simulink model "sma_corr"  (Simulink 8.0)
settings: Solver=VariableStepDiscrete  SolverMode=SingleTasking  StartTime=0.0  StopTime=1000  RelTol=1e-3
PreLoadFcn:
  | % Set this to 1 if simulating
  | simulating = 0;
  | 
  | % Overall parameters
  | n_comp = 128;
  | 
  | % Simulation
  | if (simulating)
  |     n_tvg_frames = 4;
  |     frames_per_vector = 4*8;
  |     vectors_per_wstep = 2;
  |     total_wsteps = 1; % 2^n
  |     % Laura's params
  |     x_ctr = 12;
  |     x_data_ctr = x_ctr-5;
  |     rx_addr_ctr = x_data_ctr+2;
  |     tx_addr_ctr = x_data_ctr+2;
  | else
  |     n_tvg_frames = 256;
  |     frames_per_vector = 256*8;
  |     vectors_per_wstep = 11;
  |     total_wsteps = 6; % 2^n
  |     % Laura's params
  |     x_ctr = 32;
  |     x_data_ctr = 18;
  |     rx_addr_ctr = x_data_ctr+2;
  |     tx_addr_ctr = x_data_ctr+2;
  | end
  | 
  | sb_states = repmat([ones(1, 256) zeros(1, 256)], 1, 2^(total_wsteps-1));
InitFcn:
  | % Get the XSG object to find the clock source
  | xps_xsg_blks = find_system(gcs,'FollowLinks','on','LookUnderMasks','all', 'SearchDepth',1, 'Tag','xps:xsg');
  | xsg_obj = xps_block(xps_xsg_blks{1}, {});
  | xsg_obj = xps_xsg(xsg_obj);
  | clk_src = get(xsg_obj, 'clk_src');
  | if strcmp(clk_src, 'adc0_clk')
  |     adc_brd = 'ZDOK 0';
  | elseif strcmp(clk_src, 'adc1_clk')
  |     adc_brd = 'ZDOK 1';
  | else
  |     adc_brd = '';
  | end
  | 
  | adc_clk_rate = 0;
  | % Get the appropriate ADC5g object
  | xps_adc5g_blks = find_system(gcs, 'FollowLinks', 'on', 'LookUnderMasks','all', 'SearchDepth',2, 'Tag','xps:adc5g');
  | for n = 1:length(xps_adc5g_blks)
  |     adc5g_blk = xps_adc5g_blks{n};
  |     if strcmp(get_param(adc5g_blk, 'adc_brd'), adc_brd)
  |         adc_clk_rate = 2*str2num(get_param(adc5g_blk, 'adc_clk_rate'));
  |     end
  | end
undefined symbols (not defined in the file or in its folder): Down, sfp, Up, X, xlipgetpartsetting, Z
libraries: xbsIndex_r4 x9231, casper_library_misc x178, dspsigops x32, casper_library_delays x26, xps_library x21
(part 8 of 92)

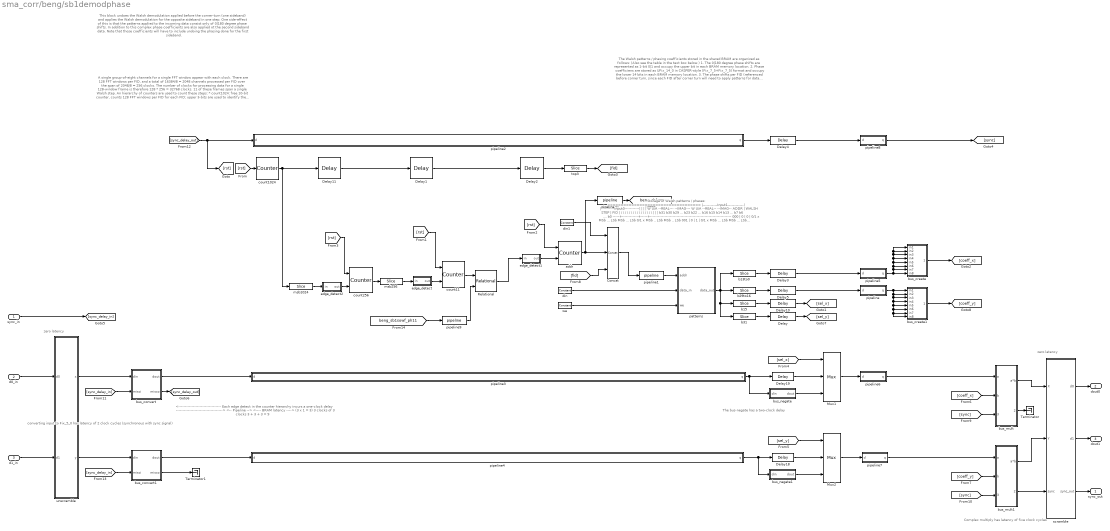
== system sma_corr/beng/sb1demodphase ==
  note: Storage of Walsh patterns / phases:
      | ===================================
      |
      |                           |-----------Input1------------| |----------Input0-----------|
      |      |            |     |  W  U/A  --REAL---   --IMAG---   W  U/A  --REAL--   --IMAG--
      | ADDR | WALSH STEP | FID | / \ / \ /         \ /         \ / \ / \ /        \ /        \
      |      |            |     | b31 b30 b29 ... b23 b22 ... b16 b15 b14 b13 ... b7 b6 .... b0
      | -----+------------+-----+-------------------------------------------------------------
      |  000 |          0 |   0 | 0/1   x MSb ... LSb MSb ... LSb 0/1   x MSb .. LSb MSb .. LSb
      |  001 |          0 |   1 | 0/1   x MSb ... LSb MSb ... LSb 0/1   x MSb .. LSb MSb .. LSb
      |    : |          : |   : |   :   :      :           :       :    :      :          :
      |  007 |          0 |   7 | 0/1   x MSb ... LSb MSb ... LSb 0/1   x MSb .. LSb MSb .. LSb
      |  008 |          1 |   0 | 0/1   x MSb ... LSb MSb ... LSb 0/1   x MSb .. LSb MSb .. LSb
      |  009 |          1 |   2 | 0/1   x MSb ... LSb MSb ... LSb 0/1   x MSb .. LSb MSb .. LSb
      |    : |          : |   : |   :   :      :           :       :    :      :          :
      |  1FF |         63 |   7 | 0/1   x MSb ... LSb MSb ... LSb 0/1   x MSb .. LSb MSb .. LSb
      |  200 |        N/A | N/A |   x   x      x           x       x    x      x          x
      |  201 |        N/A | N/A |   x   x      x           x       x    x      x          x
      |    : |          : |   : |   :   :      :           :       :    :      :          :
      |  3FF |        N/A | N/A |   x   x      x           x       x    x      x          x
  note: A single group-of-eight channels for a single FFT window appear with each clock. There are 128 FFT windows per FID,
      | and a total of 16384/8 = 2048 channels processed per FID over the span of 2048/8 = 256 clocks. The number of
      | clocks for processing data for a single 128-window frame is therefore 128 * 256 = 32768 clocks. 11 of these frames
      | span a single Walsh step. An hierarchy of counters are used to count these steps:
      |
      | 	* count1024: free 10-bit counter, counts 128 FFT windows per FID for each FID; upper 3-bits are used to identify
      | 	the current FID to select the correct bit from the loaded Walsh pattern
      |
      | 	* count256: free 8-bit counter, increments for each wrap of count1024; counts 256 group-of-eights, so when this
      | 	counter wraps, a complete 128-window frame has been processed
      |
      | 	* count11: count-limited 4-bit counter, increments for each wrap of count256; counts the 11 frames that span a
      | 	single Walsh step
      |
      | 	* addr: free 6-bit counter, increments for each wrap of count11; counts the Walsh steps in a Walsh frame; the
      | 	output is used to address the current Walsh phase that need to be applied to all FIDs.
  note: The Walsh patterns / phasing coefficients stored in the shared BRAM are organized as follows:
      | (Also see the table in the text box below.)
      |
      | 	1. The 0|180 degree phase shifts are represented as 1-bit 0|1 and occupy the upper bit in
      | 	each BRAM memory location.
      |
      | 	2. Phase coefficiens are stored as UFix_14_0 in CASPER-style (Fix_7_5+Fix_7_5) format and
      | 	occupy the lower 14 bits in each BRAM memory location.
      |
      | 	3. The phase shifts per FID (referenced before corner turn, since each FID after corner turn
      | 	will need to apply patterns for data digitized and demodulated in ALL FIDs before corner
      | 	turn) for a single Walsh step is stored in 8 consecutive shared BRAM entries along with the
      | 	phasing coefficients for each FID.
      |
      | 	4. The patterns for 64 Walsh steps are stored in sequentially blocks of eight addresses in
      | 	BRAM, so that the patterns occupy the upper bits in 64x8 = 512 (0x000-0x1FF) memory
      | 	locations. The phasing coefficients stored in memory should repeat with every Walsh step
      | 	so constant phasing is applied over the length of a complete Walsh frame.
      |
      | 	5. Due to toolflow constraints a	shared BRAM with 16-bit data has to have an address width
      | 	of at least 11 bits so the upper three quarters of memoryspace is unused.
  note: This block undoes the Walsh demodulation applied before the corner-turn (one sideband) and
      | applies the Walsh demodulation for the opposite sideband in one step. One side-effect of this
      | is that the patterns applied to the incoming data consist only of 0|180 degree phase shifts.
      |
      | In addition to this complex phase coefficients are also applied ot the second sideband data.
      | Note that those coefficients will have to include undoing the phasing done for the first
      | sideband.
  note: <-------------------------------- Each edge detect in the counter hierarchy incurs a one-clock delay -----------------------------------> <-- Pipeline --> <---- BRAM latency ---->
      |                                                     (3 x 1 = 3)                                                                               3 clocks           of 3 clocks
      |                                                            3                                                                             +        3       +           3
      |                                                          = 9
  note: The bus-negate has a
      | two-clock delay
  note: Complex multiply has latency
      | of five clock  cycles
  note: converting input to Fix_5_0 has latency
      | of 2 clock cycles (synchronous with sync
      | signal)
  note: zero latency
  note: zero latency
  sync_in : Inport
  d0_in : Inport  Port=2
  d1_in : Inport  Port=3
  Concat : xbsIndex_r4/Concat  num_inputs=3  dbl_ovrd=off  block_type=concat
  Delay{,3..5,10,18..19} (7 alike) : xbsIndex_r4/Delay  rst=off  infoeditControl=Selection of Reset will increase slice count due to use of real FFs and instead of SRLs  en=off  latency=2  dbl_ovrd=off  reg_retiming=off  block_type=delay
  Delay1 : xbsIndex_r4/Delay  rst=off  infoeditControl=Selection of Reset will increase slice count due to use of real FFs and instead of SRLs  en=off  latency=1  dbl_ovrd=off  reg_retiming=off  block_type=delay
  Delay11 : xbsIndex_r4/Delay  rst=off  infoeditControl=Selection of Reset will increase slice count due to use of real FFs and instead of SRLs  en=off  latency=1  dbl_ovrd=off  reg_retiming=off  block_type=delay
  Delay2 : xbsIndex_r4/Delay  rst=off  infoeditControl=Selection of Reset will increase slice count due to use of real FFs and instead of SRLs  en=off  latency=1  dbl_ovrd=off  reg_retiming=off  block_type=delay
  Mux1 : xbsIndex_r4/Mux  inputs=2  en=off  latency=0  precision=Full  arith_type=Unsigned  n_bits=16  bin_pt=14  quantization=Truncate  overflow=Wrap  dbl_ovrd=off  block_type=mux
  Mux2 : xbsIndex_r4/Mux  inputs=2  en=off  latency=0  precision=Full  arith_type=Unsigned  n_bits=16  bin_pt=14  quantization=Truncate  overflow=Wrap  dbl_ovrd=off  block_type=mux
  Relational : xbsIndex_r4/Relational  mode=a=b  en=off  latency=0  dbl_ovrd=off  block_type=relational
  Terminator : Terminator
  Terminator1 : Terminator
  addr : xbsIndex_r4/Counter  cnt_type=Free Running  cnt_to=Inf  operation=Up  start_count=0  cnt_by_val=1  arith_type=Unsigned  n_bits=6  bin_pt=0  load_pin=off  rst=on  en=on  explicit_period=on  period=1  dbl_ovrd=off  use_behavioral_HDL=off  implementation=Fabric  block_type=counter
  b13to0 : xbsIndex_r4/Slice  nbits=14  boolean_output=off  mode=Lower Bit Location + Width  bit1=16  base1=LSB of Input  bit0=0  base0=LSB of Input  dbl_ovrd=off  block_type=slice
  b15 : xbsIndex_r4/Slice  nbits=1  boolean_output=off  mode=Lower Bit Location + Width  bit1=0  base1=MSB of Input  bit0=15  base0=LSB of Input  dbl_ovrd=off  block_type=slice
  b29to16 : xbsIndex_r4/Slice  nbits=14  boolean_output=off  mode=Lower Bit Location + Width  bit1=0  base1=MSB of Input  bit0=16  base0=LSB of Input  dbl_ovrd=off  block_type=slice
  b31 : xbsIndex_r4/Slice  nbits=1  boolean_output=off  mode=Lower Bit Location + Width  bit1=0  base1=MSB of Input  bit0=31  base0=LSB of Input  dbl_ovrd=off  block_type=slice
  bus_convert : Subsystem  AncestorBlock=casper_library_bus/bus_convert  mask(bus_convert){n_bits_in=[4 4 4 4 4 4 4 4], bin_pt_in=[0 0 0 0 0 0 0 0], cmplx=on, n_bits_out=[5 5 5 5 5 5 5 5], bin_pt_out=[0 0 0 0 0 0 0 0], quantization=1, overflow=1, latency=2, of=off, misc=on}
      mask prompts: n_bits_in "input bit widths"; bin_pt_in "input binary points"; cmplx "input complex"; n_bits_out "output bit widths"; bin_pt_out "output binary points"; quantization "quantization strategy (Truncate=0, Round  (unbiased: +/- Inf)=1, Round (unbiased: Even Values)=2)"; overflow "overflow strategy (Wrap=0, Saturate=1, Flag as error=2)"; of "overflow indication"; misc "misc support"
      mask help: Convert components of busses
      mask init: same as sma_corr/fengine_0/cnv
  bus_convert1 : Subsystem  AncestorBlock=casper_library_bus/bus_convert  mask(bus_convert){n_bits_in=[4 4 4 4 4 4 4 4], bin_pt_in=[0 0 0 0 0 0 0 0], cmplx=on, n_bits_out=[5 5 5 5 5 5 5 5], bin_pt_out=[0 0 0 0 0 0 0 0], quantization=1, overflow=1, latency=2, of=off, misc=on}
      mask prompts: n_bits_in "input bit widths"; bin_pt_in "input binary points"; cmplx "input complex"; n_bits_out "output bit widths"; bin_pt_out "output binary points"; quantization "quantization strategy (Truncate=0, Round  (unbiased: +/- Inf)=1, Round (unbiased: Even Values)=2)"; overflow "overflow strategy (Wrap=0, Saturate=1, Flag as error=2)"; of "overflow indication"; misc "misc support"
      mask help: Convert components of busses
      mask init: same as sma_corr/fengine_0/cnv
  bus_create : Subsystem  AncestorBlock=casper_library_flow_control/bus_create  mask(bus_create){inputNum=8}
      mask prompts: inputNum "Number of inputs:"
      mask init:
      | bus_create_init(gcb,...
      |     'inputNum', inputNum);
  bus_create1 : Subsystem  AncestorBlock=casper_library_flow_control/bus_create  mask(bus_create){inputNum=8}
      mask prompts: inputNum "Number of inputs:"
      mask init:
      | bus_create_init(gcb,...
      |     'inputNum', inputNum);
  bus_mult : Subsystem  AncestorBlock=casper_library_bus/bus_mult  mask(bus_mult){n_bits_a=[5 5 5 5 5 5 5 5], bin_pt_a=[0 0 0 0 0 0 0 0], type_a=[1 1 1 1 1 1 1 1], cmplx_a=on, n_bits_b=[7 7 7 7 7 7 7 7], bin_pt_b=[5 5 5 5 5 5 5 5], type_b=[1 1 1 1 1 1 1 1], cmplx_b=on, n_bits_out=[1 1 1 1 1 1 1 1]*8, bin_pt_out=[1 1 1 1 1 1 1 1]*4, type_out=[1 1 1 1 1 1 1 1], quantization=0, overflow=1, mult_latency=3, add_latency=1, conv_latency=1, max_fanout=2, fan_latency=0, multiplier_implementation=standard core, misc=on}
      mask prompts: n_bits_a "a input bit widths"; bin_pt_a "a input binary points"; type_a "a input type (Unsigned=0, Signed=1)"; cmplx_a "a input complex"; n_bits_b "b input bit widths"; bin_pt_b "b input binary points"; type_b "b input type (Unsigned=0, Signed=1)"; cmplx_b "b input complex"; n_bits_out "output bit widths"; bin_pt_out "output binary points"; type_out "output type (Unsigned=0, Signed=1)"; quantization "quantization strategy (Truncate=0, Round  (unbiased: +/- Inf)=1)"; overflow "overflow strategy (Wrap=0, Saturate=1, Flag as error=2)"; mult_latency "multiplier latency"; add_latency "adder latency"; conv_latency "convert latency"; max_fanout "limit fanout to ?"; fan_latency "fanout register latency"; multiplier_implementation "multiplier implementation"; misc "misc support"
      mask help: Multiply components of two busses
      mask init:
      | bus_mult_init(gcb, ...
      |     'n_bits_a', n_bits_a, ...
      |     'bin_pt_a', bin_pt_a, ...
      |     'type_a', type_a, ...
      |     'cmplx_a', cmplx_a, ...
      |     'n_bits_b', n_bits_b, ...
      |     'bin_pt_b', bin_pt_b, ...
      |     'type_b', type_b, ...
      |     'cmplx_b', cmplx_b, ...
      |     'n_bits_out', n_bits_out, ...
      |     'bin_pt_out', bin_pt_out, ...
      |     'type_out', type_out, ...
      |     'quantization', quantization, ...
      |     'overflow', overflow, ...
      |     'mult_latency', mult_latency, ...
      |     'add_latency', add_latency, ...
      |     'conv_latency', conv_latency, ...
      |     'max_fanout', max_fanout, ...
      |     'fan_latency', fan_latency, ...
      |     'multiplier_implementation', multiplier_implementation, ...
      |     'misc', misc);
      mask TypeOptions multiplier_implementation: behavioral HDL | standard core | embedded multiplier core
  bus_mult1 : Subsystem  AncestorBlock=casper_library_bus/bus_mult  mask(bus_mult){n_bits_a=[5 5 5 5 5 5 5 5], bin_pt_a=[0 0 0 0 0 0 0 0], type_a=[1 1 1 1 1 1 1 1], cmplx_a=on, n_bits_b=[7 7 7 7 7 7 7 7], bin_pt_b=[5 5 5 5 5 5 5 5], type_b=[1 1 1 1 1 1 1 1], cmplx_b=on, n_bits_out=[1 1 1 1 1 1 1 1]*8, bin_pt_out=[1 1 1 1 1 1 1 1]*4, type_out=[1 1 1 1 1 1 1 1], quantization=0, overflow=1, mult_latency=3, add_latency=1, conv_latency=1, max_fanout=2, fan_latency=0, multiplier_implementation=standard core, misc=on}
      mask prompts: n_bits_a "a input bit widths"; bin_pt_a "a input binary points"; type_a "a input type (Unsigned=0, Signed=1)"; cmplx_a "a input complex"; n_bits_b "b input bit widths"; bin_pt_b "b input binary points"; type_b "b input type (Unsigned=0, Signed=1)"; cmplx_b "b input complex"; n_bits_out "output bit widths"; bin_pt_out "output binary points"; type_out "output type (Unsigned=0, Signed=1)"; quantization "quantization strategy (Truncate=0, Round  (unbiased: +/- Inf)=1)"; overflow "overflow strategy (Wrap=0, Saturate=1, Flag as error=2)"; mult_latency "multiplier latency"; add_latency "adder latency"; conv_latency "convert latency"; max_fanout "limit fanout to ?"; fan_latency "fanout register latency"; multiplier_implementation "multiplier implementation"; misc "misc support"
      mask help: Multiply components of two busses
      mask init: same as sma_corr/beng/sb1demodphase/bus_mult
      mask TypeOptions multiplier_implementation: behavioral HDL | standard core | embedded multiplier core
  bus_negate : Subsystem  AncestorBlock=casper_library_bus/bus_negate  mask(bus_negate){n_bits_in=[5 5 5 5 5 5 5 5], bin_pt_in=0, cmplx=on, overflow=1, latency=2, misc=off}
      mask prompts: n_bits_in "input bit widths"; bin_pt_in "input binary points"; cmplx "input complex"; overflow "overflow strategy (Wrap=0, Saturate=1, Flag as error=2)"; misc "misc support"
      mask help: Negate components of busses
      mask init:
      | bus_negate_init(gcb, ...
      |     'n_bits_in', n_bits_in, ...
      |     'bin_pt_in', bin_pt_in, ...
      |     'cmplx', cmplx, ...
      |     'overflow', overflow, ...
      |     'latency', latency, ...
      |     'misc', misc);
  bus_negate1 : Subsystem  AncestorBlock=casper_library_bus/bus_negate  mask(bus_negate){n_bits_in=[5 5 5 5 5 5 5 5], bin_pt_in=0, cmplx=on, overflow=1, latency=2, misc=off}
      mask prompts: n_bits_in "input bit widths"; bin_pt_in "input binary points"; cmplx "input complex"; overflow "overflow strategy (Wrap=0, Saturate=1, Flag as error=2)"; misc "misc support"
      mask help: Negate components of busses
      mask init:
      | bus_negate_init(gcb, ...
      |     'n_bits_in', n_bits_in, ...
      |     'bin_pt_in', bin_pt_in, ...
      |     'cmplx', cmplx, ...
      |     'overflow', overflow, ...
      |     'latency', latency, ...
      |     'misc', misc);
  count1024 : xbsIndex_r4/Counter  cnt_type=Free Running  cnt_to=Inf  operation=Up  start_count=0  cnt_by_val=1  arith_type=Unsigned  n_bits=10  bin_pt=0  load_pin=off  rst=on  en=off  explicit_period=on  period=1  dbl_ovrd=off  use_behavioral_HDL=off  implementation=Fabric  block_type=counter
  count11 : xbsIndex_r4/Counter  cnt_type=Count Limited  cnt_to=10  operation=Up  start_count=0  cnt_by_val=1  arith_type=Unsigned  n_bits=4  bin_pt=0  load_pin=off  rst=on  en=on  explicit_period=on  period=1  dbl_ovrd=off  use_behavioral_HDL=off  implementation=Fabric  block_type=counter
  count256 : xbsIndex_r4/Counter  cnt_type=Free Running  cnt_to=10  operation=Up  start_count=0  cnt_by_val=1  arith_type=Unsigned  n_bits=8  bin_pt=0  load_pin=off  rst=on  en=on  explicit_period=on  period=1  dbl_ovrd=off  use_behavioral_HDL=off  implementation=Fabric  block_type=counter
  din : xbsIndex_r4/Constant  const=0  gui_display_data_type=Fixed-point  arith_type=Unsigned  n_bits=8  bin_pt=0  preci_type=Single  exp_width=8  frac_width=24  explicit_period=on  period=1  equ=P=C  opselect=C  inp2=PCIN>>17  opr=+  inp1=P  carry=CIN  dbl_ovrd=off  block_type=constant
  din1 : xbsIndex_r4/Constant  const=0  gui_display_data_type=Fixed-point  arith_type=Unsigned  n_bits=1  bin_pt=0  preci_type=Single  exp_width=8  frac_width=24  explicit_period=on  period=1  equ=P=C  opselect=C  inp2=PCIN>>17  opr=+  inp1=P  carry=CIN  dbl_ovrd=off  block_type=constant
  edge_detect : Subsystem  AncestorBlock=casper_library_misc/edge_detect  mask(edge_detect){edge=Falling, polarity=Active High, x_in=[0 0.08 0.08 0.16 0.16 0.24 0.24 0.32 0.32 0.4], y_in=[-1 -1  1  1  1  1  1  1  1  1], x_out=[0.6 0.68 0.68 0.76 0.76 0.84 0.84 0.92 0.92 1], y_out=[-1 -1  1  1 -1 -1 -1 -1 -1 -1]}
      mask prompts: edge "Edge Type"; polarity "Output Polarity"; x_in "Input X Positions"; y_in "Input Y Positions"; x_out "Output X Positions"; y_out "Output Y Positions"
      mask help: Output is active for one-cycle after each edge of the specified type (i.e. rising, falling or both).
      mask init: same as sma_corr/scope/edge_detect
      settings: AttributesFormatString=|
      | falling edges
      | active high
      mask Help: Output is active for one-cycle after each edge of the specified type (i.e. rising, falling, or both).  Latency is zero.
      mask TypeOptions edge: Rising | Falling | Both
      mask TypeOptions polarity: Active High | Active Low
      mask Display:
      | plot(x_in_num,0.5+y_in_num*0.2);
      | plot(x_out_num,0.5+y_out_num*0.2);
  edge_detect1 : Subsystem  AncestorBlock=casper_library_misc/edge_detect  mask(edge_detect){edge=Falling, polarity=Active High, x_in=[0 0.08 0.08 0.16 0.16 0.24 0.24 0.32 0.32 0.4], y_in=[-1 -1  1  1  1  1  1  1  1  1], x_out=[0.6 0.68 0.68 0.76 0.76 0.84 0.84 0.92 0.92 1], y_out=[-1 -1  1  1 -1 -1 -1 -1 -1 -1]}
      mask prompts: edge "Edge Type"; polarity "Output Polarity"; x_in "Input X Positions"; y_in "Input Y Positions"; x_out "Output X Positions"; y_out "Output Y Positions"
      mask help: Output is active for one-cycle after each edge of the specified type (i.e. rising, falling or both).
      mask init: same as sma_corr/scope/edge_detect
      settings: AttributesFormatString=|
      | falling edges
      | active high
      mask Help: Output is active for one-cycle after each edge of the specified type (i.e. rising, falling, or both).  Latency is zero.
      mask TypeOptions edge: Rising | Falling | Both
      mask TypeOptions polarity: Active High | Active Low
      mask Display:
      | plot(x_in_num,0.5+y_in_num*0.2);
      | plot(x_out_num,0.5+y_out_num*0.2);
  edge_detect2 : Subsystem  AncestorBlock=casper_library_misc/edge_detect  mask(edge_detect){edge=Falling, polarity=Active High, x_in=[0 0.08 0.08 0.16 0.16 0.24 0.24 0.32 0.32 0.4], y_in=[-1 -1  1  1  1  1  1  1  1  1], x_out=[0.6 0.68 0.68 0.76 0.76 0.84 0.84 0.92 0.92 1], y_out=[-1 -1  1  1 -1 -1 -1 -1 -1 -1]}
      mask prompts: edge "Edge Type"; polarity "Output Polarity"; x_in "Input X Positions"; y_in "Input Y Positions"; x_out "Output X Positions"; y_out "Output Y Positions"
      mask help: Output is active for one-cycle after each edge of the specified type (i.e. rising, falling or both).
      mask init: same as sma_corr/scope/edge_detect
      settings: AttributesFormatString=|
      | falling edges
      | active high
      mask Help: Output is active for one-cycle after each edge of the specified type (i.e. rising, falling, or both).  Latency is zero.
      mask TypeOptions edge: Rising | Falling | Both
      mask TypeOptions polarity: Active High | Active Low
      mask Display:
      | plot(x_in_num,0.5+y_in_num*0.2);
      | plot(x_out_num,0.5+y_out_num*0.2);
  msb1024 : xbsIndex_r4/Slice  nbits=1  boolean_output=on  mode=Upper Bit Location + Width  bit1=0  base1=MSB of Input  bit0=0  base0=LSB of Input  dbl_ovrd=off  block_type=slice
  msb256 : xbsIndex_r4/Slice  nbits=1  boolean_output=on  mode=Upper Bit Location + Width  bit1=0  base1=MSB of Input  bit0=0  base0=LSB of Input  dbl_ovrd=off  block_type=slice
  patterns : Subsystem  Tag=xps:bram  AncestorBlock=xps_library/Shared BRAM  mask{arith_type=Unsigned, addr_width=10, data_width=32, reg_prim_output=on, reg_core_output=on, optimization=Minimum_Area, data_bin_pt=0, init_vals=ones(1,1024)*2101248, sample_rate=1}
      mask prompts: arith_type "Output Data Type"; addr_width "Address width"; data_width "Data Width"; reg_prim_output "Register Primitive Output"; reg_core_output "Register Core Output"; data_bin_pt "Data Binary Point"; init_vals "Initial values (simulation only)"; sample_rate "Sample rate"
      mask init:
      | shared_bram_mask;
      mask TypeOptions arith_type: Unsigned | Signed  (2's comp)
      mask TypeOptions data_width: 8 | 16 | 32 | 64 | 128
      mask TypeOptions optimization: Minimum_Area | Low_Power
  pipeline : Subsystem  AncestorBlock=casper_library_delays/pipeline  mask(pipeline){latency=5}
      mask help:
      | An explicitly laid-out delay line to avoid mapping to SRL16s
      | for use in pipelining to help achieve timing closure.
      mask init:
      | pipeline_init(gcb,'latency',latency);
      settings: AttributesFormatString=Latency = %<latency>
      mask Display:
      | port_label('input', 1, 'd');
      | port_label('output', 1, 'q');
      | disp(['Z^-', num2str(latency)]);
  pipeline1 : casper_library_delays/pipeline  latency=3
      settings: AttributesFormatString=Latency = %<latency>
  pipeline10 : casper_library_delays/pipeline  latency=3
      settings: AttributesFormatString=Latency = %<latency>
  pipeline2 : Subsystem  AncestorBlock=casper_library_delays/pipeline  mask(pipeline){latency=9}
      mask help:
      | An explicitly laid-out delay line to avoid mapping to SRL16s
      | for use in pipelining to help achieve timing closure.
      mask init:
      | pipeline_init(gcb,'latency',latency);
      settings: AttributesFormatString=Latency = %<latency>
      mask Display:
      | port_label('input', 1, 'd');
      | port_label('output', 1, 'q');
      | disp(['Z^-', num2str(latency)]);
  pipeline3 : Subsystem  AncestorBlock=casper_library_delays/pipeline  mask(pipeline){latency=9}
      mask help:
      | An explicitly laid-out delay line to avoid mapping to SRL16s
      | for use in pipelining to help achieve timing closure.
      mask init:
      | pipeline_init(gcb,'latency',latency);
      settings: AttributesFormatString=Latency = %<latency>
      mask Display:
      | port_label('input', 1, 'd');
      | port_label('output', 1, 'q');
      | disp(['Z^-', num2str(latency)]);
  pipeline4 : Subsystem  AncestorBlock=casper_library_delays/pipeline  mask(pipeline){latency=9}
      mask help:
      | An explicitly laid-out delay line to avoid mapping to SRL16s
      | for use in pipelining to help achieve timing closure.
      mask init:
      | pipeline_init(gcb,'latency',latency);
      settings: AttributesFormatString=Latency = %<latency>
      mask Display:
      | port_label('input', 1, 'd');
      | port_label('output', 1, 'q');
      | disp(['Z^-', num2str(latency)]);
  pipeline5 : Subsystem  AncestorBlock=casper_library_delays/pipeline  mask(pipeline){latency=5}
      mask help:
      | An explicitly laid-out delay line to avoid mapping to SRL16s
      | for use in pipelining to help achieve timing closure.
      mask init:
      | pipeline_init(gcb,'latency',latency);
      settings: AttributesFormatString=Latency = %<latency>
      mask Display:
      | port_label('input', 1, 'd');
      | port_label('output', 1, 'q');
      | disp(['Z^-', num2str(latency)]);
  pipeline6 : Subsystem  AncestorBlock=casper_library_delays/pipeline  mask(pipeline){latency=5}
      mask help:
      | An explicitly laid-out delay line to avoid mapping to SRL16s
      | for use in pipelining to help achieve timing closure.
      mask init:
      | pipeline_init(gcb,'latency',latency);
      settings: AttributesFormatString=Latency = %<latency>
      mask Display:
      | port_label('input', 1, 'd');
      | port_label('output', 1, 'q');
      | disp(['Z^-', num2str(latency)]);
  pipeline7 : Subsystem  AncestorBlock=casper_library_delays/pipeline  mask(pipeline){latency=5}
      mask help:
      | An explicitly laid-out delay line to avoid mapping to SRL16s
      | for use in pipelining to help achieve timing closure.
      mask init:
      | pipeline_init(gcb,'latency',latency);
      settings: AttributesFormatString=Latency = %<latency>
      mask Display:
      | port_label('input', 1, 'd');
      | port_label('output', 1, 'q');
      | disp(['Z^-', num2str(latency)]);
  pipeline8 : Subsystem  AncestorBlock=casper_library_delays/pipeline  mask(pipeline){latency=5}
      mask help:
      | An explicitly laid-out delay line to avoid mapping to SRL16s
      | for use in pipelining to help achieve timing closure.
      mask init:
      | pipeline_init(gcb,'latency',latency);
      settings: AttributesFormatString=Latency = %<latency>
      mask Display:
      | port_label('input', 1, 'd');
      | port_label('output', 1, 'q');
      | disp(['Z^-', num2str(latency)]);
  pipeline9 : casper_library_delays/pipeline  latency=3
      settings: AttributesFormatString=Latency = %<latency>
  scramble : Subsystem
  top3 : xbsIndex_r4/Slice  nbits=3  boolean_output=off  mode=Upper Bit Location + Width  bit1=0  base1=MSB of Input  bit0=0  base0=LSB of Input  dbl_ovrd=off  block_type=slice
  unscramble : Subsystem
  we : xbsIndex_r4/Constant  const=0  gui_display_data_type=Fixed-point  arith_type=Unsigned  n_bits=8  bin_pt=0  preci_type=Single  exp_width=8  frac_width=24  explicit_period=on  period=1  equ=P=C  opselect=C  inp2=PCIN>>17  opr=+  inp1=P  carry=CIN  dbl_ovrd=off  block_type=constant
  sync_out : Outport
  dout0 : Outport  Port=2
  dout1 : Outport  Port=3
  tag scope: beng_sb1ws=global
  wires:
  bus_negate/1(dout) -> Mux1/3
  Delay19/1 -> Mux1/2
  b15/1 -> Delay10/1
  patterns/1(data_out) -> b13to0/1, b29to16/1, b31/1, b15/1
  msb256/1 -> edge_detect/1(in)
  edge_detect/1(out) -> count11/2
  din/1 -> patterns/2(data_in)
  we/1 -> patterns/3(we)
  count11/1 -> Relational/1
  [rst] -> count1024/1
  [rst] -> count11/1
  [rst] -> addr/1
  Delay1/1 -> Delay2/1
  pipeline3/1(q) -> bus_negate/1(din), Delay19/1
  Relational/1 -> edge_detect1/1(in)
  edge_detect1/1(out) -> addr/2
  msb1024/1 -> edge_detect2/1(in)
  edge_detect2/1(out) -> count256/2
  [rst] -> count256/1
  count256/1 -> msb256/1
  Delay11/1 -> Delay1/1
  count1024/1 -> Delay11/1, msb1024/1
  bus_negate1/1(dout) -> Mux2/3
  Delay18/1 -> Mux2/2
  pipeline4/1(q) -> Delay18/1, bus_negate1/1(din)
  Delay10/1 -> [sel_x]
  [sel_x] -> Mux1/1
  [sel_y] -> Mux2/1
  pipeline6/1(q) -> bus_mult/1(a)
  Mux1/1 -> pipeline6/1(d)
  pipeline7/1(q) -> bus_mult1/1(a)
  Mux2/1 -> pipeline7/1(d)
  bus_mult1/2(misco) -> scramble/3(sync)
  bus_create/1(bus_out) -> [coeff_x]
  [coeff_x] -> bus_mult/2(b)
  [coeff_y] -> bus_mult1/2(b)
  Delay2/1 -> top3/1
  top3/1 -> [fid]
  [fid] -> Concat/3
  addr/1 -> pipeline10/1, Concat/2
  din1/1 -> Concat/1
  b13to0/1 -> Delay3/1
  [sync] -> bus_mult/3(misci)
  [sync] -> bus_mult1/3(misci)
  Delay3/1 -> pipeline5/1(d)
  bus_convert/1(dout) -> pipeline3/1(d)
  sync_in/1 -> [sync_delay_in]
  [sync_delay_in] -> bus_convert/2(misci)
  bus_convert/2(misco) -> [sync_delay_out]
  [sync_delay_out] -> pipeline2/1(d), [rst]
  [sync_delay_in] -> bus_convert1/2(misci)
  bus_convert1/2(misco) -> Terminator1/1
  bus_convert1/1(dout) -> pipeline4/1(d)
  pipeline1/1 -> patterns/1(addr)
  Concat/1 -> pipeline1/1
  pipeline8/1(q) -> [sync]
  pipeline2/1(q) -> Delay4/1
  Delay4/1 -> pipeline8/1(d)
  d0_in/1 -> unscramble/1(d0)
  d1_in/1 -> unscramble/2(d1)
  unscramble/2(y) -> bus_convert1/1(din)
  unscramble/1(x) -> bus_convert/1(din)
  pipeline5/1(q) -> bus_create/1(in1), bus_create/2(in2), bus_create/3(in3), bus_create/4(in4), bus_create/5(in5), bus_create/6(in6), bus_create/7(in7), bus_create/8(in8)
  Delay/1 -> [sel_y]
  b31/1 -> Delay/1
  b29to16/1 -> Delay5/1
  Delay5/1 -> pipeline/1(d)
  pipeline/1(q) -> bus_create1/2(in2), bus_create1/3(in3), bus_create1/4(in4), bus_create1/5(in5), bus_create1/6(in6), bus_create1/7(in7), bus_create1/8(in8), bus_create1/1(in1)
  bus_create1/1(bus_out) -> [coeff_y]
  bus_mult/2(misco) -> Terminator/1
  bus_mult/1(a*b) -> scramble/1(X)
  bus_mult1/1(a*b) -> scramble/2(Y)
  scramble/1(d0) -> dout0/1
  scramble/2(d1) -> dout1/1
  scramble/3(sync_out) -> sync_out/1
  [beng_sb1sowf_ph11] -> pipeline9/1
  pipeline9/1 -> Relational/2
  pipeline10/1 -> [beng_sb1ws]

== system sma_corr/beng/sum0 ==
  note: din is 64 bits wide.
      | This is eight 4+4-bit samples wide.
      | More specifically, it is 4 dual-pol
      | 4+4 bit samples:
      |
      | ||CaX|CaY||CbX|CbY||CcX|CcY||CdX|CdY||
      |
      | The four summers operate in SIMD mode
      | on four 4-bit slices of data.  We want
      | to enable/disable the two polarizations
      | indpendently so each summer must process
      | data from a single polarization only.
      |
      | The input BitBasher block slices the
      | data appropriately and the output
      | BitBasher block reassembles the data
      | back to the original layout (though
      | each sample is now wider after summing).
  gain : Inport
  sync_in : Inport  Port=2
  din : Inport  Port=3
  disable : Inport  Port=4
  BitBasher : xbsIndex_r4/BitBasher  bitexpr=|  display_expr=off  sr_1=1  arith_type1=Unsigned  bin_pt1=0  sr_2=2  arith_type2=Unsigned  bin_pt2=0  sr_3=3  arith_type3=Unsigned  bin_pt3=0  sr_4=4  arith_type4=Unsigned  bin_pt4=0  dbl_ovrd=off  block_type=bitbasher
      | <!DOCTYPE HTML PUBLIC "-//W3C//DTD HTML 4.0//EN" "http://www.w3.org/TR/REC-html40/strict.dtd">
      | <html><head><meta name="qrichtext" content="1" /><style type="text/css">
      | p, li { white-space: pre-wrap; }
      | </style></head><body style=" font-family:'Sans'; font-size:10pt; font-weight:400; font-style:normal;">
      | <p style=" margin-top:0px; margin-bottom:0px; margin-left:0px; margin-right:0px; -qt-block-indent:0; text-indent:0px;"><span style=" font-family:'MS Shell Dlg'; font-size:8pt;">Xab={i[63:56], i[47:40]}<br />Xcd={i[31:24], i[15:8]}<br />Yab={i[55:48], i[39:32]}<br />Ycd={i[23:16], i[7:0]}<br /></span></p></body></html>
  BitBasher1 : xbsIndex_r4/BitBasher  bitexpr=|  display_expr=off  sr_1=1  arith_type1=Unsigned  bin_pt1=4  sr_2=2  arith_type2=Unsigned  bin_pt2=4  sr_3=3  arith_type3=Unsigned  bin_pt3=0  sr_4=4  arith_type4=Unsigned  bin_pt4=0  dbl_ovrd=off  block_type=bitbasher
      | <!DOCTYPE HTML PUBLIC "-//W3C//DTD HTML 4.0//EN" "http://www.w3.org/TR/REC-html40/strict.dtd">
      | <html><head><meta name="qrichtext" content="1" /><style type="text/css">
      | p, li { white-space: pre-wrap; }
      | </style></head><body style=" font-family:'Sans'; font-size:10pt; font-weight:400; font-style:normal;">
      | <p style=" margin-top:12px; margin-bottom:12px; margin-left:0px; margin-right:0px; -qt-block-indent:0; text-indent:0px;"><span style=" font-family:'MS Shell Dlg'; font-size:8pt;">gx={gyx[7:0]}<br />gy={gyx[15:8]}</span></p></body></html>
  BitBasher2 : xbsIndex_r4/BitBasher  bitexpr=|  display_expr=off  sr_1=1  arith_type1=Unsigned  bin_pt1=0  sr_2=2  arith_type2=Unsigned  bin_pt2=0  sr_3=3  arith_type3=Unsigned  bin_pt3=0  sr_4=4  arith_type4=Unsigned  bin_pt4=0  dbl_ovrd=off  block_type=bitbasher
      | <!DOCTYPE HTML PUBLIC "-//W3C//DTD HTML 4.0//EN" "http://www.w3.org/TR/REC-html40/strict.dtd">
      | <html><head><meta name="qrichtext" content="1" /><style type="text/css">
      | p, li { white-space: pre-wrap; }
      | </style></head><body style=" font-family:'Sans'; font-size:10pt; font-weight:400; font-style:normal;">
      | <p style=" margin-top:0px; margin-bottom:0px; margin-left:0px; margin-right:0px; -qt-block-indent:0; text-indent:0px;"><span style=" font-family:'MS Shell Dlg'; font-size:8pt;">o={Xab[15:8], Yab[15:8], Xab[7:0], Yab[7:0], Xcd[15:8], Ycd[15:8], Xcd[7:0], Ycd[7:0]}</span></p></body></html>
  BitBasher3 : xbsIndex_r4/BitBasher  bitexpr=|  display_expr=off  sr_1=1  arith_type1=Unsigned  bin_pt1=0  sr_2=2  arith_type2=Unsigned  bin_pt2=0  sr_3=3  arith_type3=Unsigned  bin_pt3=0  sr_4=4  arith_type4=Unsigned  bin_pt4=0  dbl_ovrd=off  block_type=bitbasher
      | <!DOCTYPE HTML PUBLIC "-//W3C//DTD HTML 4.0//EN" "http://www.w3.org/TR/REC-html40/strict.dtd">
      | <html><head><meta name="qrichtext" content="1" /><style type="text/css">
      | p, li { white-space: pre-wrap; }
      | </style></head><body style=" font-family:'Sans'; font-size:10pt; font-weight:400; font-style:normal;">
      | <p style=" margin-top:12px; margin-bottom:12px; margin-left:0px; margin-right:0px; -qt-block-indent:0; text-indent:0px;"><span style=" font-family:'MS Shell Dlg'; font-size:8pt;">dx={dyx[7:0]}<br />dy={dyx[15:8]}</span></p></body></html>
  sum_Xab : Subsystem
  sum_Xcd : Subsystem
  sum_Yab : Subsystem
  sum_Ycd : Subsystem
  sync_out : Outport
  valid : Outport  Port=2
  dout : Outport  Port=3
  wires:
  gain/1 -> BitBasher1/1
  sync_in/1 -> sum_Ycd/2(sync_in), sum_Yab/2(sync_in), sum_Xcd/2(sync_in), sum_Xab/2(sync_in)
  BitBasher/1 -> sum_Xab/3(in16)
  sum_Xab/3(sum16) -> BitBasher2/1
  BitBasher/2 -> sum_Xcd/3(in16)
  BitBasher/3 -> sum_Yab/3(in16)
  BitBasher/4 -> sum_Ycd/3(in16)
  BitBasher2/1 -> dout/1
  sum_Ycd/1(sum16) -> BitBasher2/4
  sum_Xcd/1(sum16) -> BitBasher2/2
  sum_Yab/1(sum16) -> BitBasher2/3
  sum_Xab/1(sync_out) -> sync_out/1
  sum_Xab/2(valid) -> valid/1
  din/1 -> BitBasher/1
  BitBasher1/1 -> sum_Xcd/1(gain), sum_Xab/1(gain)
  BitBasher1/2 -> sum_Ycd/1(gain), sum_Yab/1(gain)
  disable/1 -> BitBasher3/1
  BitBasher3/2 -> sum_Ycd/4(disable), sum_Yab/4(disable)
  BitBasher3/1 -> sum_Xcd/4(disable), sum_Xab/4(disable)

== system sma_corr/beng/sum1 ==
  note: din is 64 bits wide.
      | This is eight 4+4-bit samples wide.
      | More specifically, it is 4 dual-pol
      | 4+4 bit samples:
      |
      | ||CaX|CaY||CbX|CbY||CcX|CcY||CdX|CdY||
      |
      | The four summers operate in SIMD mode
      | on four 4-bit slices of data.  We want
      | to enable/disable the two polarizations
      | indpendently so each summer must process
      | data from a single polarization only.
      |
      | The input BitBasher block slices the
      | data appropriately and the output
      | BitBasher block reassembles the data
      | back to the original layout (though
      | each sample is now wider after summing).
  gain : Inport
  sync_in : Inport  Port=2
  din : Inport  Port=3
  disable : Inport  Port=4
  BitBasher : xbsIndex_r4/BitBasher  bitexpr=|  display_expr=off  sr_1=1  arith_type1=Unsigned  bin_pt1=0  sr_2=2  arith_type2=Unsigned  bin_pt2=0  sr_3=3  arith_type3=Unsigned  bin_pt3=0  sr_4=4  arith_type4=Unsigned  bin_pt4=0  dbl_ovrd=off  block_type=bitbasher
      | <!DOCTYPE HTML PUBLIC "-//W3C//DTD HTML 4.0//EN" "http://www.w3.org/TR/REC-html40/strict.dtd">
      | <html><head><meta name="qrichtext" content="1" /><style type="text/css">
      | p, li { white-space: pre-wrap; }
      | </style></head><body style=" font-family:'Sans'; font-size:10pt; font-weight:400; font-style:normal;">
      | <p style=" margin-top:0px; margin-bottom:0px; margin-left:0px; margin-right:0px; -qt-block-indent:0; text-indent:0px;"><span style=" font-family:'MS Shell Dlg'; font-size:8pt;">Xab={i[63:56], i[47:40]}<br />Xcd={i[31:24], i[15:8]}<br />Yab={i[55:48], i[39:32]}<br />Ycd={i[23:16], i[7:0]}<br /></span></p></body></html>
  BitBasher1 : xbsIndex_r4/BitBasher  bitexpr=|  display_expr=off  sr_1=1  arith_type1=Unsigned  bin_pt1=4  sr_2=2  arith_type2=Unsigned  bin_pt2=4  sr_3=3  arith_type3=Unsigned  bin_pt3=0  sr_4=4  arith_type4=Unsigned  bin_pt4=0  dbl_ovrd=off  block_type=bitbasher
      | <!DOCTYPE HTML PUBLIC "-//W3C//DTD HTML 4.0//EN" "http://www.w3.org/TR/REC-html40/strict.dtd">
      | <html><head><meta name="qrichtext" content="1" /><style type="text/css">
      | p, li { white-space: pre-wrap; }
      | </style></head><body style=" font-family:'Sans'; font-size:10pt; font-weight:400; font-style:normal;">
      | <p style=" margin-top:12px; margin-bottom:12px; margin-left:0px; margin-right:0px; -qt-block-indent:0; text-indent:0px;"><span style=" font-family:'MS Shell Dlg'; font-size:8pt;">gx={gyx[7:0]}<br />gy={gyx[15:8]}</span></p></body></html>
  BitBasher2 : xbsIndex_r4/BitBasher  bitexpr=|  display_expr=off  sr_1=1  arith_type1=Unsigned  bin_pt1=0  sr_2=2  arith_type2=Unsigned  bin_pt2=0  sr_3=3  arith_type3=Unsigned  bin_pt3=0  sr_4=4  arith_type4=Unsigned  bin_pt4=0  dbl_ovrd=off  block_type=bitbasher
      | <!DOCTYPE HTML PUBLIC "-//W3C//DTD HTML 4.0//EN" "http://www.w3.org/TR/REC-html40/strict.dtd">
      | <html><head><meta name="qrichtext" content="1" /><style type="text/css">
      | p, li { white-space: pre-wrap; }
      | </style></head><body style=" font-family:'Sans'; font-size:10pt; font-weight:400; font-style:normal;">
      | <p style=" margin-top:0px; margin-bottom:0px; margin-left:0px; margin-right:0px; -qt-block-indent:0; text-indent:0px;"><span style=" font-family:'MS Shell Dlg'; font-size:8pt;">o={Xab[15:8], Yab[15:8], Xab[7:0], Yab[7:0], Xcd[15:8], Ycd[15:8], Xcd[7:0], Ycd[7:0]}</span></p></body></html>
  BitBasher3 : xbsIndex_r4/BitBasher  bitexpr=|  display_expr=off  sr_1=1  arith_type1=Unsigned  bin_pt1=0  sr_2=2  arith_type2=Unsigned  bin_pt2=0  sr_3=3  arith_type3=Unsigned  bin_pt3=0  sr_4=4  arith_type4=Unsigned  bin_pt4=0  dbl_ovrd=off  block_type=bitbasher
      | <!DOCTYPE HTML PUBLIC "-//W3C//DTD HTML 4.0//EN" "http://www.w3.org/TR/REC-html40/strict.dtd">
      | <html><head><meta name="qrichtext" content="1" /><style type="text/css">
      | p, li { white-space: pre-wrap; }
      | </style></head><body style=" font-family:'Sans'; font-size:10pt; font-weight:400; font-style:normal;">
      | <p style=" margin-top:12px; margin-bottom:12px; margin-left:0px; margin-right:0px; -qt-block-indent:0; text-indent:0px;"><span style=" font-family:'MS Shell Dlg'; font-size:8pt;">dx={dyx[7:0]}<br />dy={dyx[15:8]}</span></p></body></html>
  sum_Xab : Subsystem
  sum_Xcd : Subsystem
  sum_Yab : Subsystem
  sum_Ycd : Subsystem
  sync_out : Outport
  valid : Outport  Port=2
  dout : Outport  Port=3
  wires:
  gain/1 -> BitBasher1/1
  sync_in/1 -> sum_Ycd/2(sync_in), sum_Yab/2(sync_in), sum_Xcd/2(sync_in), sum_Xab/2(sync_in)
  BitBasher/1 -> sum_Xab/3(in16)
  sum_Xab/3(sum16) -> BitBasher2/1
  BitBasher/2 -> sum_Xcd/3(in16)
  BitBasher/3 -> sum_Yab/3(in16)
  BitBasher/4 -> sum_Ycd/3(in16)
  BitBasher2/1 -> dout/1
  sum_Ycd/1(sum16) -> BitBasher2/4
  sum_Xcd/1(sum16) -> BitBasher2/2
  sum_Yab/1(sum16) -> BitBasher2/3
  sum_Xab/1(sync_out) -> sync_out/1
  sum_Xab/2(valid) -> valid/1
  din/1 -> BitBasher/1
  BitBasher1/1 -> sum_Xcd/1(gain), sum_Xab/1(gain)
  BitBasher1/2 -> sum_Ycd/1(gain), sum_Yab/1(gain)
  disable/1 -> BitBasher3/1
  BitBasher3/2 -> sum_Ycd/4(disable), sum_Yab/4(disable)
  BitBasher3/1 -> sum_Xcd/4(disable), sum_Xab/4(disable)

== system sma_corr/beng/sum2 ==
  note: din is 128 bits wide.
      | This is eight 8+8-bit samples wide.
      | More specifically, it is 4 dual-pol
      | 8+8 bit samples:
      |
      | ||CaX|CaY||CbX|CbY||CcX|CcY||CdX|CdY||
      |
      | We want to enable/disable the two polarizations
      | indpendently so each summer must process
      | data from a single polarization only.
      |
      | The input BitBasher block slices the
      | data appropriately and the output
      | BitBasher block reassembles the data
      | back to the original layout (though
      | each sample is now wider after summing).
  gain : Inport
  sync_in : Inport  Port=2
  din : Inport  Port=3
  disable : Inport  Port=4
  BitBasher : xbsIndex_r4/BitBasher  bitexpr=|  display_expr=off  sr_1=1  arith_type1=Unsigned  bin_pt1=0  sr_2=2  arith_type2=Unsigned  bin_pt2=0  sr_3=3  arith_type3=Unsigned  bin_pt3=0  sr_4=4  arith_type4=Unsigned  bin_pt4=0  dbl_ovrd=off  block_type=bitbasher
      | <!DOCTYPE HTML PUBLIC "-//W3C//DTD HTML 4.0//EN" "http://www.w3.org/TR/REC-html40/strict.dtd">
      | <html><head><meta name="qrichtext" content="1" /><style type="text/css">
      | p, li { white-space: pre-wrap; }
      | </style></head><body style=" font-family:'Ubuntu'; font-size:11pt; font-weight:400; font-style:normal;">
      | <p style=" margin-top:0px; margin-bottom:0px; margin-left:0px; margin-right:0px; -qt-block-indent:0; text-indent:0px;"><span style=" font-family:'MS Shell Dlg'; font-size:8pt;">Xab={i[127:112], i[95:80]}<br />Xcd={i[63:48], i[31:16]}<br />Yab={i[111:96], i[79:64]}<br />Ycd={i[47:32], i[15:0]}<br /></span></p></body></html>
  BitBasher1 : xbsIndex_r4/BitBasher  bitexpr=|  display_expr=off  sr_1=1  arith_type1=Unsigned  bin_pt1=4  sr_2=2  arith_type2=Unsigned  bin_pt2=4  sr_3=3  arith_type3=Unsigned  bin_pt3=0  sr_4=4  arith_type4=Unsigned  bin_pt4=0  dbl_ovrd=off  block_type=bitbasher
      | <!DOCTYPE HTML PUBLIC "-//W3C//DTD HTML 4.0//EN" "http://www.w3.org/TR/REC-html40/strict.dtd">
      | <html><head><meta name="qrichtext" content="1" /><style type="text/css">
      | p, li { white-space: pre-wrap; }
      | </style></head><body style=" font-family:'Sans'; font-size:10pt; font-weight:400; font-style:normal;">
      | <p style=" margin-top:12px; margin-bottom:12px; margin-left:0px; margin-right:0px; -qt-block-indent:0; text-indent:0px;"><span style=" font-family:'MS Shell Dlg'; font-size:8pt;">gx={gyx[7:0]}<br />gy={gyx[15:8]}</span></p></body></html>
  BitBasher2 : xbsIndex_r4/BitBasher  bitexpr=|  display_expr=off  sr_1=1  arith_type1=Unsigned  bin_pt1=0  sr_2=2  arith_type2=Unsigned  bin_pt2=0  sr_3=3  arith_type3=Unsigned  bin_pt3=0  sr_4=4  arith_type4=Unsigned  bin_pt4=0  dbl_ovrd=off  block_type=bitbasher
      | <!DOCTYPE HTML PUBLIC "-//W3C//DTD HTML 4.0//EN" "http://www.w3.org/TR/REC-html40/strict.dtd">
      | <html><head><meta name="qrichtext" content="1" /><style type="text/css">
      | p, li { white-space: pre-wrap; }
      | </style></head><body style=" font-family:'Sans'; font-size:10pt; font-weight:400; font-style:normal;">
      | <p style=" margin-top:0px; margin-bottom:0px; margin-left:0px; margin-right:0px; -qt-block-indent:0; text-indent:0px;"><span style=" font-family:'MS Shell Dlg'; font-size:8pt;">o={Xab[15:8], Yab[15:8], Xab[7:0], Yab[7:0], Xcd[15:8], Ycd[15:8], Xcd[7:0], Ycd[7:0]}</span></p></body></html>
  BitBasher3 : xbsIndex_r4/BitBasher  bitexpr=|  display_expr=off  sr_1=1  arith_type1=Unsigned  bin_pt1=0  sr_2=2  arith_type2=Unsigned  bin_pt2=0  sr_3=3  arith_type3=Unsigned  bin_pt3=0  sr_4=4  arith_type4=Unsigned  bin_pt4=0  dbl_ovrd=off  block_type=bitbasher
      | <!DOCTYPE HTML PUBLIC "-//W3C//DTD HTML 4.0//EN" "http://www.w3.org/TR/REC-html40/strict.dtd">
      | <html><head><meta name="qrichtext" content="1" /><style type="text/css">
      | p, li { white-space: pre-wrap; }
      | </style></head><body style=" font-family:'Sans'; font-size:10pt; font-weight:400; font-style:normal;">
      | <p style=" margin-top:12px; margin-bottom:12px; margin-left:0px; margin-right:0px; -qt-block-indent:0; text-indent:0px;"><span style=" font-family:'MS Shell Dlg'; font-size:8pt;">dx={dyx[7:0]}<br />dy={dyx[15:8]}</span></p></body></html>
  sum_Xab : Subsystem
  sum_Xcd : Subsystem
  sum_Yab : Subsystem
  sum_Ycd : Subsystem
  sync_out : Outport
  valid : Outport  Port=2
  dout : Outport  Port=3
  wires:
  gain/1 -> BitBasher1/1
  sync_in/1 -> sum_Xab/2(sync_in), sum_Xcd/2(sync_in), sum_Ycd/2(sync_in), sum_Yab/2(sync_in)
  BitBasher/1 -> sum_Xab/3(in32)
  sum_Xab/3(sum16) -> BitBasher2/1
  BitBasher/2 -> sum_Xcd/3(in32)
  BitBasher/3 -> sum_Yab/3(in32)
  BitBasher/4 -> sum_Ycd/3(in32)
  sum_Xab/1(sync_out) -> sync_out/1
  sum_Xab/2(valid) -> valid/1
  din/1 -> BitBasher/1
  BitBasher1/1 -> sum_Xcd/1(gain), sum_Xab/1(gain)
  BitBasher1/2 -> sum_Ycd/1(gain), sum_Yab/1(gain)
  disable/1 -> BitBasher3/1
  BitBasher3/2 -> sum_Ycd/4(disable), sum_Yab/4(disable)
  BitBasher3/1 -> sum_Xcd/4(disable), sum_Xab/4(disable)
  sum_Ycd/3(sum16) -> BitBasher2/4
  sum_Yab/3(sum16) -> BitBasher2/3
  sum_Xcd/3(sum16) -> BitBasher2/2
  BitBasher2/1 -> dout/1
